simulink model "l8_ver130"  (Simulink 7.9)
settings: Solver=FixedStepDiscrete  SolverMode=SingleTasking  StartTime=0.0  StopTime=10  RelTol=1e-3
undefined symbols (not defined in the file or in its folder): sfp
libraries: xbsIndex_r4 x20466, casper_library_misc x43, dspsigops x32, simulink x7, xps_library x5, casper_library_flow_control x4
(part 22 of 210)

== system l8_ver130/s2/lo_6 ==
  addr : Inport
  pol1 : Inport  Port=2
  pol2 : Inport  Port=3
  Constant10 : xbsIndex_r4/Constant  const=0  gui_display_data_type=Fixed-point  arith_type=Unsigned  n_bits=18  bin_pt=0  preci_type=Single  exp_width=8  frac_width=24  explicit_period=on  period=1  equ=P=C  opselect=C  inp2=PCIN>>17  opr=+  inp1=P  carry=CIN  dbl_ovrd=off  block_type=constant
  Constant9 : xbsIndex_r4/Constant  const=0  gui_display_data_type=Fixed-point  arith_type=Unsigned  n_bits=18  bin_pt=0  preci_type=Single  exp_width=8  frac_width=24  explicit_period=on  period=1  equ=P=C  opselect=C  inp2=PCIN>>17  opr=+  inp1=P  carry=CIN  dbl_ovrd=off  block_type=constant
  Gateway Out : xbsIndex_r4/Gateway Out  inherit_from_input=off  hdl_port=on  timing_constraint=None  locs_specified=off  LOCs={}  UseAsDAC=off  DACChannel='1'  block_type=gatewayout
      settings: infoedit="Gateway out block.  Converts Xilinx fixed point or floating-point type inputs into ouputs of type Simulink integer, double, or fixed point.<br><br>Hardware notes:  In hardware these blocks become top level output ports or are discarded, depending on how they are configured."
  Gateway Out1 : xbsIndex_r4/Gateway Out  inherit_from_input=off  hdl_port=on  timing_constraint=None  locs_specified=off  LOCs={}  UseAsDAC=off  DACChannel='1'  block_type=gatewayout
      settings: infoedit="Gateway out block.  Converts Xilinx fixed point or floating-point type inputs into ouputs of type Simulink integer, double, or fixed point.<br><br>Hardware notes:  In hardware these blocks become top level output ports or are discarded, depending on how they are configured."
  Mult{1..3,16} (4 alike) : xbsIndex_r4/Mult  precision=Full  arith_type="Signed  (2's comp)"  n_bits=16  bin_pt=14  quantization=Truncate  overflow=Wrap  en=off  latency=mult_latency  dbl_ovrd=off  use_behavioral_HDL=off  opt=Speed  use_embedded=on  optimum_pipeline=off  pipeline=on  use_rpm=on  placement_style=Triangular  block_type=mult
      settings: block_version=11.4
  Reinterpret12 : xbsIndex_r4/Reinterpret  force_arith_type=on  arith_type="Signed  (2's comp)"  force_bin_pt=on  bin_pt=15  block_type=reinterpret
  Reinterpret9 : xbsIndex_r4/Reinterpret  force_arith_type=on  arith_type="Signed  (2's comp)"  force_bin_pt=on  bin_pt=15  block_type=reinterpret
      settings: infoedit="Changes signal type without altering the binary representation.   You can changed the signal between signed and unsigned, and relocate the binary point.<br><br>Hardware notes: In hardware this block costs nothing.<br><br>Example:  Suppose the input is 6 bits wide, signed, with 2 fractional bits, and the output is forced to unsigned with 0 fractional bits.  Then an input of -2.0 (1110.00 in binary 2's complement) becomes an output of 56 (111000 in binary)."
  Scope : Scope  NumInputPorts=2
      settings: YMin=-0.0075~-1  YMax=0.0075~1  SaveName=ScopeData3
  cos_slice : xbsIndex_r4/Slice  nbits=16  boolean_output=off  mode=Lower Bit Location + Width  bit1=0  base1=MSB of Input  bit0=0  base0=LSB of Input  dbl_ovrd=off  block_type=slice
  delay{1..3,80} (4 alike) : xbsIndex_r4/Delay  rst=off  infoeditControl=Selection of Reset will increase slice count due to use of real FFs and instead of SRLs  en=off  latency=3  dbl_ovrd=off  reg_retiming=on  block_type=delay
      settings: block_version=11.4
  delay{4..10,32} (8 alike) : xbsIndex_r4/Delay  rst=off  infoeditControl=Selection of Reset will increase slice count due to use of real FFs and instead of SRLs  en=off  latency=4  dbl_ovrd=off  reg_retiming=on  block_type=delay
      settings: block_version=11.4
  lo_ram : Subsystem  Tag=xps:bram  AncestorBlock=xps_library/Shared BRAM  mask{arith_type=Signed  (2's comp), addr_width=bram_length, data_width=32, reg_prim_output=off, reg_core_output=off, optimization=Minimum_Area, data_bin_pt=0, init_vals=[1:2^11], sample_rate=1}
      mask prompts: arith_type "Output Data Type"; addr_width "Address width"; data_width "Data Width"; reg_prim_output "Register Primitive Output"; reg_core_output "Register Core Output"; data_bin_pt "Data Binary Point"; init_vals "Initial values (simulation only)"; sample_rate "Sample rate"
      mask init:
      | shared_bram_mask;
      mask StyleString: popup(Unsigned|Signed  (2's comp)),edit,popup(8|16|32|64|128),checkbox,checkbox,popup(Minimum_Area|Low_Power),edit,edit,edit
  sin_slice : xbsIndex_r4/Slice  nbits=16  boolean_output=off  mode=Upper Bit Location + Width  bit1=0  base1=MSB of Input  bit0=0  base0=LSB of Input  dbl_ovrd=off  block_type=slice
  pol1_im : Outport
  pol2_im : Outport  Port=2
  pol1_re : Outport  Port=3
  pol2_re : Outport  Port=4
  wires:
  Constant9/1 -> lo_ram/3(we)
  Constant10/1 -> lo_ram/2(data_in)
  Mult16/1 -> delay80/1
  Reinterpret12/1 -> Gateway Out/1, delay6/1, delay4/1
  sin_slice/1 -> Reinterpret12/1
  lo_ram/1(data_out) -> cos_slice/1, sin_slice/1
  Mult1/1 -> delay1/1
  Mult2/1 -> delay2/1
  Reinterpret9/1 -> Gateway Out1/1, delay10/1, delay8/1
  cos_slice/1 -> Reinterpret9/1
  Mult3/1 -> delay3/1
  delay32/1 -> Mult16/1
  delay4/1 -> Mult16/2
  delay5/1 -> Mult1/1
  delay6/1 -> Mult1/2
  delay7/1 -> Mult2/1
  delay8/1 -> Mult2/2
  delay9/1 -> Mult3/1
  delay10/1 -> Mult3/2
  delay80/1 -> pol1_im/1
  pol2/1 -> delay5/1, delay9/1
  delay1/1 -> pol2_im/1
  delay2/1 -> pol1_re/1
  delay3/1 -> pol2_re/1
  pol1/1 -> delay32/1, delay7/1
  addr/1 -> lo_ram/1(addr)
  Gateway Out/1 -> Scope/1
  Gateway Out1/1 -> Scope/2

== system l8_ver130/s2/lo_7 ==
  addr : Inport
  pol1 : Inport  Port=2
  pol2 : Inport  Port=3
  Constant10 : xbsIndex_r4/Constant  const=0  gui_display_data_type=Fixed-point  arith_type=Unsigned  n_bits=18  bin_pt=0  preci_type=Single  exp_width=8  frac_width=24  explicit_period=on  period=1  equ=P=C  opselect=C  inp2=PCIN>>17  opr=+  inp1=P  carry=CIN  dbl_ovrd=off  block_type=constant
  Constant9 : xbsIndex_r4/Constant  const=0  gui_display_data_type=Fixed-point  arith_type=Unsigned  n_bits=18  bin_pt=0  preci_type=Single  exp_width=8  frac_width=24  explicit_period=on  period=1  equ=P=C  opselect=C  inp2=PCIN>>17  opr=+  inp1=P  carry=CIN  dbl_ovrd=off  block_type=constant
  Gateway Out : xbsIndex_r4/Gateway Out  inherit_from_input=off  hdl_port=on  timing_constraint=None  locs_specified=off  LOCs={}  UseAsDAC=off  DACChannel='1'  block_type=gatewayout
      settings: infoedit="Gateway out block.  Converts Xilinx fixed point or floating-point type inputs into ouputs of type Simulink integer, double, or fixed point.<br><br>Hardware notes:  In hardware these blocks become top level output ports or are discarded, depending on how they are configured."
  Gateway Out1 : xbsIndex_r4/Gateway Out  inherit_from_input=off  hdl_port=on  timing_constraint=None  locs_specified=off  LOCs={}  UseAsDAC=off  DACChannel='1'  block_type=gatewayout
      settings: infoedit="Gateway out block.  Converts Xilinx fixed point or floating-point type inputs into ouputs of type Simulink integer, double, or fixed point.<br><br>Hardware notes:  In hardware these blocks become top level output ports or are discarded, depending on how they are configured."
  Mult{1..3,16} (4 alike) : xbsIndex_r4/Mult  precision=Full  arith_type="Signed  (2's comp)"  n_bits=16  bin_pt=14  quantization=Truncate  overflow=Wrap  en=off  latency=mult_latency  dbl_ovrd=off  use_behavioral_HDL=off  opt=Speed  use_embedded=on  optimum_pipeline=off  pipeline=on  use_rpm=on  placement_style=Triangular  block_type=mult
      settings: block_version=11.4
  Reinterpret12 : xbsIndex_r4/Reinterpret  force_arith_type=on  arith_type="Signed  (2's comp)"  force_bin_pt=on  bin_pt=15  block_type=reinterpret
  Reinterpret9 : xbsIndex_r4/Reinterpret  force_arith_type=on  arith_type="Signed  (2's comp)"  force_bin_pt=on  bin_pt=15  block_type=reinterpret
      settings: infoedit="Changes signal type without altering the binary representation.   You can changed the signal between signed and unsigned, and relocate the binary point.<br><br>Hardware notes: In hardware this block costs nothing.<br><br>Example:  Suppose the input is 6 bits wide, signed, with 2 fractional bits, and the output is forced to unsigned with 0 fractional bits.  Then an input of -2.0 (1110.00 in binary 2's complement) becomes an output of 56 (111000 in binary)."
  Scope : Scope  NumInputPorts=2
      settings: YMin=-0.0075~-1  YMax=0.0075~1  SaveName=ScopeData3
  cos_slice : xbsIndex_r4/Slice  nbits=16  boolean_output=off  mode=Lower Bit Location + Width  bit1=0  base1=MSB of Input  bit0=0  base0=LSB of Input  dbl_ovrd=off  block_type=slice
  delay{1..3,80} (4 alike) : xbsIndex_r4/Delay  rst=off  infoeditControl=Selection of Reset will increase slice count due to use of real FFs and instead of SRLs  en=off  latency=3  dbl_ovrd=off  reg_retiming=on  block_type=delay
      settings: block_version=11.4
  delay{4..10,32} (8 alike) : xbsIndex_r4/Delay  rst=off  infoeditControl=Selection of Reset will increase slice count due to use of real FFs and instead of SRLs  en=off  latency=4  dbl_ovrd=off  reg_retiming=on  block_type=delay
      settings: block_version=11.4
  lo_ram : Subsystem  Tag=xps:bram  AncestorBlock=xps_library/Shared BRAM  mask{arith_type=Signed  (2's comp), addr_width=bram_length, data_width=32, reg_prim_output=off, reg_core_output=off, optimization=Minimum_Area, data_bin_pt=0, init_vals=[1:2^11], sample_rate=1}
      mask prompts: arith_type "Output Data Type"; addr_width "Address width"; data_width "Data Width"; reg_prim_output "Register Primitive Output"; reg_core_output "Register Core Output"; data_bin_pt "Data Binary Point"; init_vals "Initial values (simulation only)"; sample_rate "Sample rate"
      mask init:
      | shared_bram_mask;
      mask StyleString: popup(Unsigned|Signed  (2's comp)),edit,popup(8|16|32|64|128),checkbox,checkbox,popup(Minimum_Area|Low_Power),edit,edit,edit
  sin_slice : xbsIndex_r4/Slice  nbits=16  boolean_output=off  mode=Upper Bit Location + Width  bit1=0  base1=MSB of Input  bit0=0  base0=LSB of Input  dbl_ovrd=off  block_type=slice
  pol1_im : Outport
  pol2_im : Outport  Port=2
  pol1_re : Outport  Port=3
  pol2_re : Outport  Port=4
  wires:
  Gateway Out1/1 -> Scope/2
  Gateway Out/1 -> Scope/1
  addr/1 -> lo_ram/1(addr)
  pol1/1 -> delay7/1, delay32/1
  delay3/1 -> pol2_re/1
  delay2/1 -> pol1_re/1
  delay1/1 -> pol2_im/1
  pol2/1 -> delay9/1, delay5/1
  delay80/1 -> pol1_im/1
  delay10/1 -> Mult3/2
  delay9/1 -> Mult3/1
  delay8/1 -> Mult2/2
  delay7/1 -> Mult2/1
  delay6/1 -> Mult1/2
  delay5/1 -> Mult1/1
  delay4/1 -> Mult16/2
  delay32/1 -> Mult16/1
  Mult3/1 -> delay3/1
  cos_slice/1 -> Reinterpret9/1
  Reinterpret9/1 -> delay8/1, delay10/1, Gateway Out1/1
  Mult2/1 -> delay2/1
  Mult1/1 -> delay1/1
  lo_ram/1(data_out) -> sin_slice/1, cos_slice/1
  sin_slice/1 -> Reinterpret12/1
  Reinterpret12/1 -> delay4/1, delay6/1, Gateway Out/1
  Mult16/1 -> delay80/1
  Constant10/1 -> lo_ram/2(data_in)
  Constant9/1 -> lo_ram/3(we)

== system l8_ver130/s2/lo_8 ==
  addr : Inport
  pol1 : Inport  Port=2
  pol2 : Inport  Port=3
  Constant10 : xbsIndex_r4/Constant  const=0  gui_display_data_type=Fixed-point  arith_type=Unsigned  n_bits=18  bin_pt=0  preci_type=Single  exp_width=8  frac_width=24  explicit_period=on  period=1  equ=P=C  opselect=C  inp2=PCIN>>17  opr=+  inp1=P  carry=CIN  dbl_ovrd=off  block_type=constant
  Constant9 : xbsIndex_r4/Constant  const=0  gui_display_data_type=Fixed-point  arith_type=Unsigned  n_bits=18  bin_pt=0  preci_type=Single  exp_width=8  frac_width=24  explicit_period=on  period=1  equ=P=C  opselect=C  inp2=PCIN>>17  opr=+  inp1=P  carry=CIN  dbl_ovrd=off  block_type=constant
  Gateway Out : xbsIndex_r4/Gateway Out  inherit_from_input=off  hdl_port=on  timing_constraint=None  locs_specified=off  LOCs={}  UseAsDAC=off  DACChannel='1'  block_type=gatewayout
      settings: infoedit="Gateway out block.  Converts Xilinx fixed point or floating-point type inputs into ouputs of type Simulink integer, double, or fixed point.<br><br>Hardware notes:  In hardware these blocks become top level output ports or are discarded, depending on how they are configured."
  Gateway Out1 : xbsIndex_r4/Gateway Out  inherit_from_input=off  hdl_port=on  timing_constraint=None  locs_specified=off  LOCs={}  UseAsDAC=off  DACChannel='1'  block_type=gatewayout
      settings: infoedit="Gateway out block.  Converts Xilinx fixed point or floating-point type inputs into ouputs of type Simulink integer, double, or fixed point.<br><br>Hardware notes:  In hardware these blocks become top level output ports or are discarded, depending on how they are configured."
  Mult{1..3,16} (4 alike) : xbsIndex_r4/Mult  precision=Full  arith_type="Signed  (2's comp)"  n_bits=16  bin_pt=14  quantization=Truncate  overflow=Wrap  en=off  latency=mult_latency  dbl_ovrd=off  use_behavioral_HDL=off  opt=Speed  use_embedded=on  optimum_pipeline=off  pipeline=on  use_rpm=on  placement_style=Triangular  block_type=mult
      settings: block_version=11.4
  Reinterpret12 : xbsIndex_r4/Reinterpret  force_arith_type=on  arith_type="Signed  (2's comp)"  force_bin_pt=on  bin_pt=15  block_type=reinterpret
  Reinterpret9 : xbsIndex_r4/Reinterpret  force_arith_type=on  arith_type="Signed  (2's comp)"  force_bin_pt=on  bin_pt=15  block_type=reinterpret
      settings: infoedit="Changes signal type without altering the binary representation.   You can changed the signal between signed and unsigned, and relocate the binary point.<br><br>Hardware notes: In hardware this block costs nothing.<br><br>Example:  Suppose the input is 6 bits wide, signed, with 2 fractional bits, and the output is forced to unsigned with 0 fractional bits.  Then an input of -2.0 (1110.00 in binary 2's complement) becomes an output of 56 (111000 in binary)."
  Scope : Scope  NumInputPorts=2
      settings: YMin=-0.0075~-1  YMax=0.0075~1  SaveName=ScopeData3
  cos_slice : xbsIndex_r4/Slice  nbits=16  boolean_output=off  mode=Lower Bit Location + Width  bit1=0  base1=MSB of Input  bit0=0  base0=LSB of Input  dbl_ovrd=off  block_type=slice
  delay{1..3,80} (4 alike) : xbsIndex_r4/Delay  rst=off  infoeditControl=Selection of Reset will increase slice count due to use of real FFs and instead of SRLs  en=off  latency=3  dbl_ovrd=off  reg_retiming=on  block_type=delay
      settings: block_version=11.4
  delay{4..10,32} (8 alike) : xbsIndex_r4/Delay  rst=off  infoeditControl=Selection of Reset will increase slice count due to use of real FFs and instead of SRLs  en=off  latency=4  dbl_ovrd=off  reg_retiming=on  block_type=delay
      settings: block_version=11.4
  lo_ram : Subsystem  Tag=xps:bram  AncestorBlock=xps_library/Shared BRAM  mask{arith_type=Signed  (2's comp), addr_width=bram_length, data_width=32, reg_prim_output=off, reg_core_output=off, optimization=Minimum_Area, data_bin_pt=0, init_vals=[1:2^11], sample_rate=1}
      mask prompts: arith_type "Output Data Type"; addr_width "Address width"; data_width "Data Width"; reg_prim_output "Register Primitive Output"; reg_core_output "Register Core Output"; data_bin_pt "Data Binary Point"; init_vals "Initial values (simulation only)"; sample_rate "Sample rate"
      mask init:
      | shared_bram_mask;
      mask StyleString: popup(Unsigned|Signed  (2's comp)),edit,popup(8|16|32|64|128),checkbox,checkbox,popup(Minimum_Area|Low_Power),edit,edit,edit
  sin_slice : xbsIndex_r4/Slice  nbits=16  boolean_output=off  mode=Upper Bit Location + Width  bit1=0  base1=MSB of Input  bit0=0  base0=LSB of Input  dbl_ovrd=off  block_type=slice
  pol1_im : Outport
  pol2_im : Outport  Port=2
  pol1_re : Outport  Port=3
  pol2_re : Outport  Port=4
  wires:
  Constant9/1 -> lo_ram/3(we)
  Constant10/1 -> lo_ram/2(data_in)
  Mult16/1 -> delay80/1
  Reinterpret12/1 -> Gateway Out/1, delay6/1, delay4/1
  sin_slice/1 -> Reinterpret12/1
  lo_ram/1(data_out) -> cos_slice/1, sin_slice/1
  Mult1/1 -> delay1/1
  Mult2/1 -> delay2/1
  Reinterpret9/1 -> Gateway Out1/1, delay10/1, delay8/1
  cos_slice/1 -> Reinterpret9/1
  Mult3/1 -> delay3/1
  delay32/1 -> Mult16/1
  delay4/1 -> Mult16/2
  delay5/1 -> Mult1/1
  delay6/1 -> Mult1/2
  delay7/1 -> Mult2/1
  delay8/1 -> Mult2/2
  delay9/1 -> Mult3/1
  delay10/1 -> Mult3/2
  delay80/1 -> pol1_im/1
  pol2/1 -> delay5/1, delay9/1
  delay1/1 -> pol2_im/1
  delay2/1 -> pol1_re/1
  delay3/1 -> pol2_re/1
  pol1/1 -> delay32/1, delay7/1
  addr/1 -> lo_ram/1(addr)
  Gateway Out/1 -> Scope/1
  Gateway Out1/1 -> Scope/2

== system l8_ver130/s2/lo_9 ==
  addr : Inport
  pol1 : Inport  Port=2
  pol2 : Inport  Port=3
  Constant10 : xbsIndex_r4/Constant  const=0  gui_display_data_type=Fixed-point  arith_type=Unsigned  n_bits=18  bin_pt=0  preci_type=Single  exp_width=8  frac_width=24  explicit_period=on  period=1  equ=P=C  opselect=C  inp2=PCIN>>17  opr=+  inp1=P  carry=CIN  dbl_ovrd=off  block_type=constant
  Constant9 : xbsIndex_r4/Constant  const=0  gui_display_data_type=Fixed-point  arith_type=Unsigned  n_bits=18  bin_pt=0  preci_type=Single  exp_width=8  frac_width=24  explicit_period=on  period=1  equ=P=C  opselect=C  inp2=PCIN>>17  opr=+  inp1=P  carry=CIN  dbl_ovrd=off  block_type=constant
  Gateway Out : xbsIndex_r4/Gateway Out  inherit_from_input=off  hdl_port=on  timing_constraint=None  locs_specified=off  LOCs={}  UseAsDAC=off  DACChannel='1'  block_type=gatewayout
      settings: infoedit="Gateway out block.  Converts Xilinx fixed point or floating-point type inputs into ouputs of type Simulink integer, double, or fixed point.<br><br>Hardware notes:  In hardware these blocks become top level output ports or are discarded, depending on how they are configured."
  Gateway Out1 : xbsIndex_r4/Gateway Out  inherit_from_input=off  hdl_port=on  timing_constraint=None  locs_specified=off  LOCs={}  UseAsDAC=off  DACChannel='1'  block_type=gatewayout
      settings: infoedit="Gateway out block.  Converts Xilinx fixed point or floating-point type inputs into ouputs of type Simulink integer, double, or fixed point.<br><br>Hardware notes:  In hardware these blocks become top level output ports or are discarded, depending on how they are configured."
  Mult{1..3,16} (4 alike) : xbsIndex_r4/Mult  precision=Full  arith_type="Signed  (2's comp)"  n_bits=16  bin_pt=14  quantization=Truncate  overflow=Wrap  en=off  latency=mult_latency  dbl_ovrd=off  use_behavioral_HDL=off  opt=Speed  use_embedded=on  optimum_pipeline=off  pipeline=on  use_rpm=on  placement_style=Triangular  block_type=mult
      settings: block_version=11.4
  Reinterpret12 : xbsIndex_r4/Reinterpret  force_arith_type=on  arith_type="Signed  (2's comp)"  force_bin_pt=on  bin_pt=15  block_type=reinterpret
  Reinterpret9 : xbsIndex_r4/Reinterpret  force_arith_type=on  arith_type="Signed  (2's comp)"  force_bin_pt=on  bin_pt=15  block_type=reinterpret
      settings: infoedit="Changes signal type without altering the binary representation.   You can changed the signal between signed and unsigned, and relocate the binary point.<br><br>Hardware notes: In hardware this block costs nothing.<br><br>Example:  Suppose the input is 6 bits wide, signed, with 2 fractional bits, and the output is forced to unsigned with 0 fractional bits.  Then an input of -2.0 (1110.00 in binary 2's complement) becomes an output of 56 (111000 in binary)."
  Scope : Scope  NumInputPorts=2
      settings: YMin=-0.0075~-1  YMax=0.0075~1  SaveName=ScopeData3
  cos_slice : xbsIndex_r4/Slice  nbits=16  boolean_output=off  mode=Lower Bit Location + Width  bit1=0  base1=MSB of Input  bit0=0  base0=LSB of Input  dbl_ovrd=off  block_type=slice
  delay{1..3,80} (4 alike) : xbsIndex_r4/Delay  rst=off  infoeditControl=Selection of Reset will increase slice count due to use of real FFs and instead of SRLs  en=off  latency=3  dbl_ovrd=off  reg_retiming=on  block_type=delay
      settings: block_version=11.4
  delay{4..10,32} (8 alike) : xbsIndex_r4/Delay  rst=off  infoeditControl=Selection of Reset will increase slice count due to use of real FFs and instead of SRLs  en=off  latency=4  dbl_ovrd=off  reg_retiming=on  block_type=delay
      settings: block_version=11.4
  lo_ram : Subsystem  Tag=xps:bram  AncestorBlock=xps_library/Shared BRAM  mask{arith_type=Signed  (2's comp), addr_width=bram_length, data_width=32, reg_prim_output=off, reg_core_output=off, optimization=Minimum_Area, data_bin_pt=0, init_vals=[1:2^11], sample_rate=1}
      mask prompts: arith_type "Output Data Type"; addr_width "Address width"; data_width "Data Width"; reg_prim_output "Register Primitive Output"; reg_core_output "Register Core Output"; data_bin_pt "Data Binary Point"; init_vals "Initial values (simulation only)"; sample_rate "Sample rate"
      mask init:
      | shared_bram_mask;
      mask StyleString: popup(Unsigned|Signed  (2's comp)),edit,popup(8|16|32|64|128),checkbox,checkbox,popup(Minimum_Area|Low_Power),edit,edit,edit
  sin_slice : xbsIndex_r4/Slice  nbits=16  boolean_output=off  mode=Upper Bit Location + Width  bit1=0  base1=MSB of Input  bit0=0  base0=LSB of Input  dbl_ovrd=off  block_type=slice
  pol1_im : Outport
  pol2_im : Outport  Port=2
  pol1_re : Outport  Port=3
  pol2_re : Outport  Port=4
  wires:
  Gateway Out1/1 -> Scope/2
  Gateway Out/1 -> Scope/1
  addr/1 -> lo_ram/1(addr)
  pol1/1 -> delay7/1, delay32/1
  delay3/1 -> pol2_re/1
  delay2/1 -> pol1_re/1
  delay1/1 -> pol2_im/1
  pol2/1 -> delay9/1, delay5/1
  delay80/1 -> pol1_im/1
  delay10/1 -> Mult3/2
  delay9/1 -> Mult3/1
  delay8/1 -> Mult2/2
  delay7/1 -> Mult2/1
  delay6/1 -> Mult1/2
  delay5/1 -> Mult1/1
  delay4/1 -> Mult16/2
  delay32/1 -> Mult16/1
  Mult3/1 -> delay3/1
  cos_slice/1 -> Reinterpret9/1
  Reinterpret9/1 -> delay8/1, delay10/1, Gateway Out1/1
  Mult2/1 -> delay2/1
  Mult1/1 -> delay1/1
  lo_ram/1(data_out) -> sin_slice/1, cos_slice/1
  sin_slice/1 -> Reinterpret12/1
  Reinterpret12/1 -> delay4/1, delay6/1, Gateway Out/1
  Mult16/1 -> delay80/1
  Constant10/1 -> lo_ram/2(data_in)
  Constant9/1 -> lo_ram/3(we)

== system l8_ver130/s2/mixer_cnt ==
  sim_reg : Inport
  io_delay : xbsIndex_r4/Delay  rst=off  infoeditControl=Selection of Reset will increase slice count due to use of real FFs and instead of SRLs  en=off  latency=0  dbl_ovrd=off  reg_retiming=on  block_type=delay
  l8_ver130_s2_mixer_cnt_user_data_out : xbsIndex_r4/Gateway In  gui_display_data_type=Fixed-point  arith_type=Unsigned  n_bits=32  bin_pt=0  preci_type=Single  exp_width=8  frac_width=24  quantization="Round  (unbiased: +/- Inf)"  overflow=Saturate  period=1  dbl_ovrd=off  timing_constraint=None  locs_specified=off  LOCs={}  UseAsADC=off  ADCChannel='1'  inherit_from_input=off  hdl_port=on  block_type=gatewayin
  reint1 : xbsIndex_r4/Reinterpret  force_arith_type=on  arith_type=Unsigned  force_bin_pt=on  bin_pt=0  block_type=reinterpret
  slice_reg : xbsIndex_r4/Slice  nbits=32  boolean_output=off  mode=Lower Bit Location + Width  bit1=0  base1=MSB of Input  bit0=0  base0=LSB of Input  dbl_ovrd=off  block_type=slice
  in_reg : Outport
  wires:
  l8_ver130_s2_mixer_cnt_user_data_out/1 -> io_delay/1
  sim_reg/1 -> l8_ver130_s2_mixer_cnt_user_data_out/1
  io_delay/1 -> slice_reg/1
  slice_reg/1 -> reint1/1
  reint1/1 -> in_reg/1

== system l8_ver130/s2/quant ==
  In2 : Inport
  In3 : Inport  Port=2
  In4 : Inport  Port=3
  In5 : Inport  Port=4
  Constant : Constant
  Delay{,2,4,6,8} (5 alike) : xbsIndex_r4/Delay  rst=off  infoeditControl=Selection of Reset will increase slice count due to use of real FFs and instead of SRLs  en=off  latency=5  dbl_ovrd=off  reg_retiming=off  block_type=delay
  Delay{1,3,5,7} (4 alike) : xbsIndex_r4/Delay  rst=off  infoeditControl=Selection of Reset will increase slice count due to use of real FFs and instead of SRLs  en=off  latency=1  dbl_ovrd=off  reg_retiming=off  block_type=delay
  gain : Subsystem  Tag=xps:sw_reg  AncestorBlock=xps_library/software register  mask{numios=1, io_dir=From Processor, io_delay=0, sample_period=1, name1=reg, bitwidth1=32, arith_type1=Unsigned, bin_pt1=0}
      mask prompts: numios "Num I/Os"; io_dir "I/O direction"; io_delay "I/O delay"; sample_period "Sample period"; name1 "Name"; bitwidth1 "Bitwidth"; arith_type1 "Type"; bin_pt1 "Binary pt"
      mask init:
      | swreg_init(gcb);
      mask StyleString: edit,popup(To Processor|From Processor),edit,edit,edit,edit,popup(Unsigned|Signed  (2's comp)|Boolean),edit
      mask CallbackString:
      | t = timer('StartDelay', 0.1); t.TimerFcn = {@swreg_cb, gcb}; start(t);|a = get_param(gcb, 'MaskEnables');
      | if strcmp(get_param(gcb, 'io_dir'), 'To Processor'),
      | a(4) = {'off'};
      | else
      | a(4) = {'on'};
      | end
      | set_param(gcb, 'MaskEnables', a);
      | |||||a = get_param(gcb, 'MaskEnables');
      | if strcmp(get_param(gcb, 'arith_type1'), 'Boolean'),
      | set_param(gcb, 'bitwidth1', '1');
      | set_param(gcb, 'bin_pt1', '0');
      | a(6) = {'off'};
      | a(8) = {'off'};
      | else
      | a(6) = {'on'};
      | a(8) = {'on'};
      | end
      | set_param(gcb, 'MaskEnables', a);
      | |
      mask TabNameString: Setup,Setup,Setup,Setup,IO1,IO1,IO1,IO1
  round : Subsystem
  round1 : Subsystem
  round2 : Subsystem
  round3 : Subsystem
  Out1 : Outport
  Out2 : Outport  Port=2
  Out3 : Outport  Port=3
  Out4 : Outport  Port=4
  wires:
  Delay1/1 -> Delay/1
  Delay3/1 -> Delay2/1
  Delay5/1 -> Delay4/1
  Delay7/1 -> Delay6/1
  Delay/1 -> round/1(dIn)
  Delay2/1 -> round1/1(dIn)
  Delay4/1 -> round2/1(dIn)
  Delay6/1 -> round3/1(dIn)
  gain/1(in_reg) -> Delay8/1
  Delay8/1 -> round/2(Scale), round1/2(Scale), round2/2(Scale), round3/2(Scale)
  In2/1 -> Delay1/1
  round/1(dOut) -> Out1/1
  In3/1 -> Delay3/1
  round1/1(dOut) -> Out2/1
  In4/1 -> Delay5/1
  round2/1(dOut) -> Out3/1
  In5/1 -> Delay7/1
  round3/1(dOut) -> Out4/1
  Constant/1 -> gain/1(sim_reg)

== system l8_ver130/s3/Halfband0 ==
  in : Inport
  sync : Inport  Port=2
  Delay{1..14} (14 alike) : xbsIndex_r4/Delay  rst=off  infoeditControl=Selection of Reset will increase slice count due to use of real FFs and instead of SRLs  en=off  latency=2  dbl_ovrd=off  reg_retiming=off  block_type=delay
  adder_tree : Subsystem
      settings: AttributesFormatString=|
      | latency 4
      | hold period:2
  en_gen : Subsystem
      settings: AttributesFormatString=|
      | hold period: 2
      | explicit clk period:off , input clk period:1
      | relational/logical delay:1
      | extra_delay:0
  hold_fir_tap1 : Subsystem
      settings: AttributesFormatString=|
      | coefficient: -0.026473
      |  hold period: 2
      |  mult latency: 3
  hold_fir_tap11 : Subsystem
      settings: AttributesFormatString=|
      | coefficient: -0.093407
      |  hold period: 2
      |  mult latency: 3
  hold_fir_tap13 : Subsystem
      settings: AttributesFormatString=|
      | coefficient: 0.044090
      |  hold period: 2
      |  mult latency: 3
  hold_fir_tap15 : Subsystem
      settings: AttributesFormatString=|
      | coefficient: -0.026473
      |  hold period: 2
      |  mult latency: 3
  hold_fir_tap3 : Subsystem
      settings: AttributesFormatString=|
      | coefficient: 0.044090
      |  hold period: 2
      |  mult latency: 3
  hold_fir_tap5 : Subsystem
      settings: AttributesFormatString=|
      | coefficient: -0.093407
      |  hold period: 2
      |  mult latency: 3
  hold_fir_tap7 : Subsystem
      settings: AttributesFormatString=|
      | coefficient: 0.313910
      |  hold period: 2
      |  mult latency: 3
  hold_fir_tap8 : Subsystem
      settings: AttributesFormatString=|
      | coefficient: 0.500000
      |  hold period: 2
      |  mult latency: 3
  hold_fir_tap9 : Subsystem
      settings: AttributesFormatString=|
      | coefficient: 0.313910
      |  hold period: 2
      |  mult latency: 3
  out : Outport
  en_out : Outport  Port=2
  wires:
  in/1 -> hold_fir_tap1/1(data_in), Delay1/1
  Delay2/1 -> hold_fir_tap3/1(data_in), Delay3/1  "xlsub2_Delay_out2"
  Delay4/1 -> hold_fir_tap5/1(data_in), Delay5/1  "xlsub2_Delay_out4"
  Delay6/1 -> hold_fir_tap7/1(data_in), Delay7/1  "xlsub2_Delay_out6"
  Delay7/1 -> hold_fir_tap8/1(data_in), Delay8/1  "xlsub2_Delay_out7"
  Delay8/1 -> hold_fir_tap9/1(data_in), Delay9/1  "xlsub2_Delay_out8"
  Delay10/1 -> hold_fir_tap11/1(data_in), Delay11/1  "xlsub2_Delay_out10"
  Delay12/1 -> hold_fir_tap13/1(data_in), Delay13/1  "xlsub2_Delay_out12"
  en_gen/1(en_out) -> adder_tree/1(en_in), hold_fir_tap15/2(en_in), hold_fir_tap13/2(en_in), hold_fir_tap11/2(en_in), hold_fir_tap9/2(en_in), hold_fir_tap8/2(en_in), hold_fir_tap7/2(en_in), hold_fir_tap5/2(en_in), hold_fir_tap3/2(en_in), hold_fir_tap1/2(en_in)  "en"
  adder_tree/2(dout) -> out/1
  adder_tree/1(en_out) -> en_out/1
  hold_fir_tap15/1(mult_out) -> adder_tree/10(din9)  "hold_fir_tap_out9"
  hold_fir_tap13/1(mult_out) -> adder_tree/9(din8)  "hold_fir_tap_out8"
  hold_fir_tap11/1(mult_out) -> adder_tree/8(din7)  "hold_fir_tap_out7"
  hold_fir_tap9/1(mult_out) -> adder_tree/7(din6)  "hold_fir_tap_out6"
  hold_fir_tap8/1(mult_out) -> adder_tree/6(din5)  "hold_fir_tap_out5"
  hold_fir_tap7/1(mult_out) -> adder_tree/5(din4)  "hold_fir_tap_out4"
  hold_fir_tap5/1(mult_out) -> adder_tree/4(din3)  "hold_fir_tap_out3"
  hold_fir_tap3/1(mult_out) -> adder_tree/3(din2)  "hold_fir_tap_out2"
  hold_fir_tap1/1(mult_out) -> adder_tree/2(din1)  "hold_fir_tap_out1"
  Delay14/1 -> hold_fir_tap15/1(data_in)  "xlsub2_Delay_out14"
  Delay13/1 -> Delay14/1  "xlsub2_Delay_out13"
  Delay11/1 -> Delay12/1  "xlsub2_Delay_out11"
  Delay9/1 -> Delay10/1  "xlsub2_Delay_out9"
  Delay5/1 -> Delay6/1  "xlsub2_Delay_out5"
  Delay3/1 -> Delay4/1  "xlsub2_Delay_out3"
  Delay1/1 -> Delay2/1  "xlsub2_Delay_out1"
  sync/1 -> en_gen/1(sync_in)

== system l8_ver130/s3/Halfband1 ==
  in : Inport
  sync : Inport  Port=2
  Delay{1..14} (14 alike) : xbsIndex_r4/Delay  rst=off  infoeditControl=Selection of Reset will increase slice count due to use of real FFs and instead of SRLs  en=off  latency=2  dbl_ovrd=off  reg_retiming=off  block_type=delay
  adder_tree : Subsystem
      settings: AttributesFormatString=|
      | latency 4
      | hold period:2
  en_gen : Subsystem
      settings: AttributesFormatString=|
      | hold period: 2
      | explicit clk period:off , input clk period:1
      | relational/logical delay:1
      | extra_delay:0
  hold_fir_tap1 : Subsystem
      settings: AttributesFormatString=|
      | coefficient: -0.026473
      |  hold period: 2
      |  mult latency: 3
  hold_fir_tap11 : Subsystem
      settings: AttributesFormatString=|
      | coefficient: -0.093407
      |  hold period: 2
      |  mult latency: 3
  hold_fir_tap13 : Subsystem
      settings: AttributesFormatString=|
      | coefficient: 0.044090
      |  hold period: 2
      |  mult latency: 3
  hold_fir_tap15 : Subsystem
      settings: AttributesFormatString=|
      | coefficient: -0.026473
      |  hold period: 2
      |  mult latency: 3
  hold_fir_tap3 : Subsystem
      settings: AttributesFormatString=|
      | coefficient: 0.044090
      |  hold period: 2
      |  mult latency: 3
  hold_fir_tap5 : Subsystem
      settings: AttributesFormatString=|
      | coefficient: -0.093407
      |  hold period: 2
      |  mult latency: 3
  hold_fir_tap7 : Subsystem
      settings: AttributesFormatString=|
      | coefficient: 0.313910
      |  hold period: 2
      |  mult latency: 3
  hold_fir_tap8 : Subsystem
      settings: AttributesFormatString=|
      | coefficient: 0.500000
      |  hold period: 2
      |  mult latency: 3
  hold_fir_tap9 : Subsystem
      settings: AttributesFormatString=|
      | coefficient: 0.313910
      |  hold period: 2
      |  mult latency: 3
  out : Outport
  en_out : Outport  Port=2
  wires:
  in/1 -> hold_fir_tap1/1(data_in), Delay1/1
  Delay2/1 -> hold_fir_tap3/1(data_in), Delay3/1  "xlsub2_Delay_out2"
  Delay4/1 -> hold_fir_tap5/1(data_in), Delay5/1  "xlsub2_Delay_out4"
  Delay6/1 -> hold_fir_tap7/1(data_in), Delay7/1  "xlsub2_Delay_out6"
  Delay7/1 -> hold_fir_tap8/1(data_in), Delay8/1  "xlsub2_Delay_out7"
  Delay8/1 -> hold_fir_tap9/1(data_in), Delay9/1  "xlsub2_Delay_out8"
  Delay10/1 -> hold_fir_tap11/1(data_in), Delay11/1  "xlsub2_Delay_out10"
  Delay12/1 -> hold_fir_tap13/1(data_in), Delay13/1  "xlsub2_Delay_out12"
  en_gen/1(en_out) -> adder_tree/1(en_in), hold_fir_tap15/2(en_in), hold_fir_tap13/2(en_in), hold_fir_tap11/2(en_in), hold_fir_tap9/2(en_in), hold_fir_tap8/2(en_in), hold_fir_tap7/2(en_in), hold_fir_tap5/2(en_in), hold_fir_tap3/2(en_in), hold_fir_tap1/2(en_in)  "en"
  adder_tree/2(dout) -> out/1
  adder_tree/1(en_out) -> en_out/1
  hold_fir_tap15/1(mult_out) -> adder_tree/10(din9)  "hold_fir_tap_out9"
  hold_fir_tap13/1(mult_out) -> adder_tree/9(din8)  "hold_fir_tap_out8"
  hold_fir_tap11/1(mult_out) -> adder_tree/8(din7)  "hold_fir_tap_out7"
  hold_fir_tap9/1(mult_out) -> adder_tree/7(din6)  "hold_fir_tap_out6"
  hold_fir_tap8/1(mult_out) -> adder_tree/6(din5)  "hold_fir_tap_out5"
  hold_fir_tap7/1(mult_out) -> adder_tree/5(din4)  "hold_fir_tap_out4"
  hold_fir_tap5/1(mult_out) -> adder_tree/4(din3)  "hold_fir_tap_out3"
  hold_fir_tap3/1(mult_out) -> adder_tree/3(din2)  "hold_fir_tap_out2"
  hold_fir_tap1/1(mult_out) -> adder_tree/2(din1)  "hold_fir_tap_out1"
  Delay14/1 -> hold_fir_tap15/1(data_in)  "xlsub2_Delay_out14"
  Delay13/1 -> Delay14/1  "xlsub2_Delay_out13"
  Delay11/1 -> Delay12/1  "xlsub2_Delay_out11"
  Delay9/1 -> Delay10/1  "xlsub2_Delay_out9"
  Delay5/1 -> Delay6/1  "xlsub2_Delay_out5"
  Delay3/1 -> Delay4/1  "xlsub2_Delay_out3"
  Delay1/1 -> Delay2/1  "xlsub2_Delay_out1"
  sync/1 -> en_gen/1(sync_in)

== system l8_ver130/s3/Halfband2 ==
  in : Inport
  sync : Inport  Port=2
  Delay{1..14} (14 alike) : xbsIndex_r4/Delay  rst=off  infoeditControl=Selection of Reset will increase slice count due to use of real FFs and instead of SRLs  en=off  latency=2  dbl_ovrd=off  reg_retiming=off  block_type=delay
  adder_tree : Subsystem
      settings: AttributesFormatString=|
      | latency 4
      | hold period:2
  en_gen : Subsystem
      settings: AttributesFormatString=|
      | hold period: 2
      | explicit clk period:off , input clk period:1
      | relational/logical delay:1
      | extra_delay:0
  hold_fir_tap1 : Subsystem
      settings: AttributesFormatString=|
      | coefficient: -0.026473
      |  hold period: 2
      |  mult latency: 3
  hold_fir_tap11 : Subsystem
      settings: AttributesFormatString=|
      | coefficient: -0.093407
      |  hold period: 2
      |  mult latency: 3
  hold_fir_tap13 : Subsystem
      settings: AttributesFormatString=|
      | coefficient: 0.044090
      |  hold period: 2
      |  mult latency: 3
  hold_fir_tap15 : Subsystem
      settings: AttributesFormatString=|
      | coefficient: -0.026473
      |  hold period: 2
      |  mult latency: 3
  hold_fir_tap3 : Subsystem
      settings: AttributesFormatString=|
      | coefficient: 0.044090
      |  hold period: 2
      |  mult latency: 3
  hold_fir_tap5 : Subsystem
      settings: AttributesFormatString=|
      | coefficient: -0.093407
      |  hold period: 2
      |  mult latency: 3
  hold_fir_tap7 : Subsystem
      settings: AttributesFormatString=|
      | coefficient: 0.313910
      |  hold period: 2
      |  mult latency: 3
  hold_fir_tap8 : Subsystem
      settings: AttributesFormatString=|
      | coefficient: 0.500000
      |  hold period: 2
      |  mult latency: 3
  hold_fir_tap9 : Subsystem
      settings: AttributesFormatString=|
      | coefficient: 0.313910
      |  hold period: 2
      |  mult latency: 3
  out : Outport
  en_out : Outport  Port=2
  wires:
  in/1 -> hold_fir_tap1/1(data_in), Delay1/1
  Delay2/1 -> hold_fir_tap3/1(data_in), Delay3/1  "xlsub2_Delay_out2"
  Delay4/1 -> hold_fir_tap5/1(data_in), Delay5/1  "xlsub2_Delay_out4"
  Delay6/1 -> hold_fir_tap7/1(data_in), Delay7/1  "xlsub2_Delay_out6"
  Delay7/1 -> hold_fir_tap8/1(data_in), Delay8/1  "xlsub2_Delay_out7"
  Delay8/1 -> hold_fir_tap9/1(data_in), Delay9/1  "xlsub2_Delay_out8"
  Delay10/1 -> hold_fir_tap11/1(data_in), Delay11/1  "xlsub2_Delay_out10"
  Delay12/1 -> hold_fir_tap13/1(data_in), Delay13/1  "xlsub2_Delay_out12"
  en_gen/1(en_out) -> adder_tree/1(en_in), hold_fir_tap15/2(en_in), hold_fir_tap13/2(en_in), hold_fir_tap11/2(en_in), hold_fir_tap9/2(en_in), hold_fir_tap8/2(en_in), hold_fir_tap7/2(en_in), hold_fir_tap5/2(en_in), hold_fir_tap3/2(en_in), hold_fir_tap1/2(en_in)  "en"
  adder_tree/2(dout) -> out/1
  adder_tree/1(en_out) -> en_out/1
  hold_fir_tap15/1(mult_out) -> adder_tree/10(din9)  "hold_fir_tap_out9"
  hold_fir_tap13/1(mult_out) -> adder_tree/9(din8)  "hold_fir_tap_out8"
  hold_fir_tap11/1(mult_out) -> adder_tree/8(din7)  "hold_fir_tap_out7"
  hold_fir_tap9/1(mult_out) -> adder_tree/7(din6)  "hold_fir_tap_out6"
  hold_fir_tap8/1(mult_out) -> adder_tree/6(din5)  "hold_fir_tap_out5"
  hold_fir_tap7/1(mult_out) -> adder_tree/5(din4)  "hold_fir_tap_out4"
  hold_fir_tap5/1(mult_out) -> adder_tree/4(din3)  "hold_fir_tap_out3"
  hold_fir_tap3/1(mult_out) -> adder_tree/3(din2)  "hold_fir_tap_out2"
  hold_fir_tap1/1(mult_out) -> adder_tree/2(din1)  "hold_fir_tap_out1"
  Delay14/1 -> hold_fir_tap15/1(data_in)  "xlsub2_Delay_out14"
  Delay13/1 -> Delay14/1  "xlsub2_Delay_out13"
  Delay11/1 -> Delay12/1  "xlsub2_Delay_out11"
  Delay9/1 -> Delay10/1  "xlsub2_Delay_out9"
  Delay5/1 -> Delay6/1  "xlsub2_Delay_out5"
  Delay3/1 -> Delay4/1  "xlsub2_Delay_out3"
  Delay1/1 -> Delay2/1  "xlsub2_Delay_out1"
  sync/1 -> en_gen/1(sync_in)

== system l8_ver130/s3/Halfband3 ==
  in : Inport
  sync : Inport  Port=2
  Delay{1..14} (14 alike) : xbsIndex_r4/Delay  rst=off  infoeditControl=Selection of Reset will increase slice count due to use of real FFs and instead of SRLs  en=off  latency=2  dbl_ovrd=off  reg_retiming=off  block_type=delay
  adder_tree : Subsystem
      settings: AttributesFormatString=|
      | latency 4
      | hold period:2
  en_gen : Subsystem
      settings: AttributesFormatString=|
      | hold period: 2
      | explicit clk period:off , input clk period:1
      | relational/logical delay:1
      | extra_delay:0
  hold_fir_tap1 : Subsystem
      settings: AttributesFormatString=|
      | coefficient: -0.026473
      |  hold period: 2
      |  mult latency: 3
  hold_fir_tap11 : Subsystem
      settings: AttributesFormatString=|
      | coefficient: -0.093407
      |  hold period: 2
      |  mult latency: 3
  hold_fir_tap13 : Subsystem
      settings: AttributesFormatString=|
      | coefficient: 0.044090
      |  hold period: 2
      |  mult latency: 3
  hold_fir_tap15 : Subsystem
      settings: AttributesFormatString=|
      | coefficient: -0.026473
      |  hold period: 2
      |  mult latency: 3
  hold_fir_tap3 : Subsystem
      settings: AttributesFormatString=|
      | coefficient: 0.044090
      |  hold period: 2
      |  mult latency: 3
  hold_fir_tap5 : Subsystem
      settings: AttributesFormatString=|
      | coefficient: -0.093407
      |  hold period: 2
      |  mult latency: 3
  hold_fir_tap7 : Subsystem
      settings: AttributesFormatString=|
      | coefficient: 0.313910
      |  hold period: 2
      |  mult latency: 3
  hold_fir_tap8 : Subsystem
      settings: AttributesFormatString=|
      | coefficient: 0.500000
      |  hold period: 2
      |  mult latency: 3
  hold_fir_tap9 : Subsystem
      settings: AttributesFormatString=|
      | coefficient: 0.313910
      |  hold period: 2
      |  mult latency: 3
  out : Outport
  en_out : Outport  Port=2
  wires:
  in/1 -> hold_fir_tap1/1(data_in), Delay1/1
  Delay2/1 -> hold_fir_tap3/1(data_in), Delay3/1  "xlsub2_Delay_out2"
  Delay4/1 -> hold_fir_tap5/1(data_in), Delay5/1  "xlsub2_Delay_out4"
  Delay6/1 -> hold_fir_tap7/1(data_in), Delay7/1  "xlsub2_Delay_out6"
  Delay7/1 -> hold_fir_tap8/1(data_in), Delay8/1  "xlsub2_Delay_out7"
  Delay8/1 -> hold_fir_tap9/1(data_in), Delay9/1  "xlsub2_Delay_out8"
  Delay10/1 -> hold_fir_tap11/1(data_in), Delay11/1  "xlsub2_Delay_out10"
  Delay12/1 -> hold_fir_tap13/1(data_in), Delay13/1  "xlsub2_Delay_out12"
  en_gen/1(en_out) -> adder_tree/1(en_in), hold_fir_tap15/2(en_in), hold_fir_tap13/2(en_in), hold_fir_tap11/2(en_in), hold_fir_tap9/2(en_in), hold_fir_tap8/2(en_in), hold_fir_tap7/2(en_in), hold_fir_tap5/2(en_in), hold_fir_tap3/2(en_in), hold_fir_tap1/2(en_in)  "en"
  adder_tree/2(dout) -> out/1
  adder_tree/1(en_out) -> en_out/1
  hold_fir_tap15/1(mult_out) -> adder_tree/10(din9)  "hold_fir_tap_out9"
  hold_fir_tap13/1(mult_out) -> adder_tree/9(din8)  "hold_fir_tap_out8"
  hold_fir_tap11/1(mult_out) -> adder_tree/8(din7)  "hold_fir_tap_out7"
  hold_fir_tap9/1(mult_out) -> adder_tree/7(din6)  "hold_fir_tap_out6"
  hold_fir_tap8/1(mult_out) -> adder_tree/6(din5)  "hold_fir_tap_out5"
  hold_fir_tap7/1(mult_out) -> adder_tree/5(din4)  "hold_fir_tap_out4"
  hold_fir_tap5/1(mult_out) -> adder_tree/4(din3)  "hold_fir_tap_out3"
  hold_fir_tap3/1(mult_out) -> adder_tree/3(din2)  "hold_fir_tap_out2"
  hold_fir_tap1/1(mult_out) -> adder_tree/2(din1)  "hold_fir_tap_out1"
  Delay14/1 -> hold_fir_tap15/1(data_in)  "xlsub2_Delay_out14"
  Delay13/1 -> Delay14/1  "xlsub2_Delay_out13"
  Delay11/1 -> Delay12/1  "xlsub2_Delay_out11"
  Delay9/1 -> Delay10/1  "xlsub2_Delay_out9"
  Delay5/1 -> Delay6/1  "xlsub2_Delay_out5"
  Delay3/1 -> Delay4/1  "xlsub2_Delay_out3"
  Delay1/1 -> Delay2/1  "xlsub2_Delay_out1"
  sync/1 -> en_gen/1(sync_in)

== system l8_ver130/s3/cic_pow2_0 ==
  in1 : Inport
  in2 : Inport  Port=2
  in3 : Inport  Port=3
  in4 : Inport  Port=4
  in5 : Inport  Port=5
  in6 : Inport  Port=6
  in7 : Inport  Port=7
  in8 : Inport  Port=8
  in9 : Inport  Port=9
  in10 : Inport  Port=10
  in11 : Inport  Port=11
  in12 : Inport  Port=12
  in13 : Inport  Port=13
  in14 : Inport  Port=14
  in15 : Inport  Port=15
  in16 : Inport  Port=16
  sync_in : Inport  Port=17
  Stage1_dec2 : Subsystem
      settings: AttributesFormatString=|
      | nonrecursive
      | 24_22_in
      | clock(actualy/effective): 1/1
  Stage2_dec2 : Subsystem
      settings: AttributesFormatString=|
      | nonrecursive
      | 28_22_in
      | clock(actualy/effective): 1/1
  Stage3_dec2 : Subsystem
      settings: AttributesFormatString=|
      | nonrecursive
      | 32_22_in
      | clock(actualy/effective): 1/1
  Stage4_dec2 : Subsystem
      settings: AttributesFormatString=|
      | nonrecursive
      | 36_22_in
      | clock(actualy/effective): 1/1
  out1 : Outport
  sync_out : Outport  Port=2
  wires:
  sync_in/1 -> Stage4_dec2/3(sync_in), Stage3_dec2/5(sync_in), Stage2_dec2/9(sync_in), Stage1_dec2/17(sync_in)
  Stage4_dec2/2(sync_out) -> sync_out/1  "stage_sync_out4"
  Stage4_dec2/1(out1) -> out1/1
  Stage3_dec2/2(out2) -> Stage4_dec2/2(in2)  "sig4_2"
  Stage3_dec2/1(out1) -> Stage4_dec2/1(in1)  "sig4_1"
  Stage2_dec2/4(out4) -> Stage3_dec2/4(in4)  "sig3_4"
  Stage2_dec2/3(out3) -> Stage3_dec2/3(in3)  "sig3_3"
  Stage2_dec2/2(out2) -> Stage3_dec2/2(in2)  "sig3_2"
  Stage2_dec2/1(out1) -> Stage3_dec2/1(in1)  "sig3_1"
  Stage1_dec2/8(out8) -> Stage2_dec2/8(in8)  "sig2_8"
  Stage1_dec2/7(out7) -> Stage2_dec2/7(in7)  "sig2_7"
  Stage1_dec2/6(out6) -> Stage2_dec2/6(in6)  "sig2_6"
  Stage1_dec2/5(out5) -> Stage2_dec2/5(in5)  "sig2_5"
  Stage1_dec2/4(out4) -> Stage2_dec2/4(in4)  "sig2_4"
  Stage1_dec2/3(out3) -> Stage2_dec2/3(in3)  "sig2_3"
  Stage1_dec2/2(out2) -> Stage2_dec2/2(in2)  "sig2_2"
  Stage1_dec2/1(out1) -> Stage2_dec2/1(in1)  "sig2_1"
  in16/1 -> Stage1_dec2/16(in16)
  in15/1 -> Stage1_dec2/15(in15)
  in14/1 -> Stage1_dec2/14(in14)
  in13/1 -> Stage1_dec2/13(in13)
  in12/1 -> Stage1_dec2/12(in12)
  in11/1 -> Stage1_dec2/11(in11)
  in10/1 -> Stage1_dec2/10(in10)
  in9/1 -> Stage1_dec2/9(in9)
  in8/1 -> Stage1_dec2/8(in8)
  in7/1 -> Stage1_dec2/7(in7)
  in6/1 -> Stage1_dec2/6(in6)
  in5/1 -> Stage1_dec2/5(in5)
  in4/1 -> Stage1_dec2/4(in4)
  in3/1 -> Stage1_dec2/3(in3)
  in2/1 -> Stage1_dec2/2(in2)
  in1/1 -> Stage1_dec2/1(in1)

== system l8_ver130/s3/cic_pow2_1 ==
  in1 : Inport
  in2 : Inport  Port=2
  in3 : Inport  Port=3
  in4 : Inport  Port=4
  in5 : Inport  Port=5
  in6 : Inport  Port=6
  in7 : Inport  Port=7
  in8 : Inport  Port=8
  in9 : Inport  Port=9
  in10 : Inport  Port=10
  in11 : Inport  Port=11
  in12 : Inport  Port=12
  in13 : Inport  Port=13
  in14 : Inport  Port=14
  in15 : Inport  Port=15
  in16 : Inport  Port=16
  sync_in : Inport  Port=17
  Stage1_dec2 : Subsystem
      settings: AttributesFormatString=|
      | nonrecursive
      | 24_22_in
      | clock(actualy/effective): 1/1
  Stage2_dec2 : Subsystem
      settings: AttributesFormatString=|
      | nonrecursive
      | 28_22_in
      | clock(actualy/effective): 1/1
  Stage3_dec2 : Subsystem
      settings: AttributesFormatString=|
      | nonrecursive
      | 32_22_in
      | clock(actualy/effective): 1/1
  Stage4_dec2 : Subsystem
      settings: AttributesFormatString=|
      | nonrecursive
      | 36_22_in
      | clock(actualy/effective): 1/1
  out1 : Outport
  sync_out : Outport  Port=2
  wires:
  sync_in/1 -> Stage4_dec2/3(sync_in), Stage3_dec2/5(sync_in), Stage2_dec2/9(sync_in), Stage1_dec2/17(sync_in)
  Stage4_dec2/2(sync_out) -> sync_out/1  "stage_sync_out4"
  Stage4_dec2/1(out1) -> out1/1
  Stage3_dec2/2(out2) -> Stage4_dec2/2(in2)  "sig4_2"
  Stage3_dec2/1(out1) -> Stage4_dec2/1(in1)  "sig4_1"
  Stage2_dec2/4(out4) -> Stage3_dec2/4(in4)  "sig3_4"
  Stage2_dec2/3(out3) -> Stage3_dec2/3(in3)  "sig3_3"
  Stage2_dec2/2(out2) -> Stage3_dec2/2(in2)  "sig3_2"
  Stage2_dec2/1(out1) -> Stage3_dec2/1(in1)  "sig3_1"
  Stage1_dec2/8(out8) -> Stage2_dec2/8(in8)  "sig2_8"
  Stage1_dec2/7(out7) -> Stage2_dec2/7(in7)  "sig2_7"
  Stage1_dec2/6(out6) -> Stage2_dec2/6(in6)  "sig2_6"
  Stage1_dec2/5(out5) -> Stage2_dec2/5(in5)  "sig2_5"
  Stage1_dec2/4(out4) -> Stage2_dec2/4(in4)  "sig2_4"
  Stage1_dec2/3(out3) -> Stage2_dec2/3(in3)  "sig2_3"
  Stage1_dec2/2(out2) -> Stage2_dec2/2(in2)  "sig2_2"
  Stage1_dec2/1(out1) -> Stage2_dec2/1(in1)  "sig2_1"
  in16/1 -> Stage1_dec2/16(in16)
  in15/1 -> Stage1_dec2/15(in15)
  in14/1 -> Stage1_dec2/14(in14)
  in13/1 -> Stage1_dec2/13(in13)
  in12/1 -> Stage1_dec2/12(in12)
  in11/1 -> Stage1_dec2/11(in11)
  in10/1 -> Stage1_dec2/10(in10)
  in9/1 -> Stage1_dec2/9(in9)
  in8/1 -> Stage1_dec2/8(in8)
  in7/1 -> Stage1_dec2/7(in7)
  in6/1 -> Stage1_dec2/6(in6)
  in5/1 -> Stage1_dec2/5(in5)
  in4/1 -> Stage1_dec2/4(in4)
  in3/1 -> Stage1_dec2/3(in3)
  in2/1 -> Stage1_dec2/2(in2)
  in1/1 -> Stage1_dec2/1(in1)

== system l8_ver130/s3/cic_pow2_2 ==
  in1 : Inport
  in2 : Inport  Port=2
  in3 : Inport  Port=3
  in4 : Inport  Port=4
  in5 : Inport  Port=5
  in6 : Inport  Port=6
  in7 : Inport  Port=7
  in8 : Inport  Port=8
  in9 : Inport  Port=9
  in10 : Inport  Port=10
  in11 : Inport  Port=11
  in12 : Inport  Port=12
  in13 : Inport  Port=13
  in14 : Inport  Port=14
  in15 : Inport  Port=15
  in16 : Inport  Port=16
  sync_in : Inport  Port=17
  Stage1_dec2 : Subsystem
      settings: AttributesFormatString=|
      | nonrecursive
      | 24_22_in
      | clock(actualy/effective): 1/1
  Stage2_dec2 : Subsystem
      settings: AttributesFormatString=|
      | nonrecursive
      | 28_22_in
      | clock(actualy/effective): 1/1
  Stage3_dec2 : Subsystem
      settings: AttributesFormatString=|
      | nonrecursive
      | 32_22_in
      | clock(actualy/effective): 1/1
  Stage4_dec2 : Subsystem
      settings: AttributesFormatString=|
      | nonrecursive
      | 36_22_in
      | clock(actualy/effective): 1/1
  out1 : Outport
  sync_out : Outport  Port=2
  wires:
  sync_in/1 -> Stage4_dec2/3(sync_in), Stage3_dec2/5(sync_in), Stage2_dec2/9(sync_in), Stage1_dec2/17(sync_in)
  Stage4_dec2/2(sync_out) -> sync_out/1  "stage_sync_out4"
  Stage4_dec2/1(out1) -> out1/1
  Stage3_dec2/2(out2) -> Stage4_dec2/2(in2)  "sig4_2"
  Stage3_dec2/1(out1) -> Stage4_dec2/1(in1)  "sig4_1"
  Stage2_dec2/4(out4) -> Stage3_dec2/4(in4)  "sig3_4"
  Stage2_dec2/3(out3) -> Stage3_dec2/3(in3)  "sig3_3"
  Stage2_dec2/2(out2) -> Stage3_dec2/2(in2)  "sig3_2"
  Stage2_dec2/1(out1) -> Stage3_dec2/1(in1)  "sig3_1"
  Stage1_dec2/8(out8) -> Stage2_dec2/8(in8)  "sig2_8"
  Stage1_dec2/7(out7) -> Stage2_dec2/7(in7)  "sig2_7"
  Stage1_dec2/6(out6) -> Stage2_dec2/6(in6)  "sig2_6"
  Stage1_dec2/5(out5) -> Stage2_dec2/5(in5)  "sig2_5"
  Stage1_dec2/4(out4) -> Stage2_dec2/4(in4)  "sig2_4"
  Stage1_dec2/3(out3) -> Stage2_dec2/3(in3)  "sig2_3"
  Stage1_dec2/2(out2) -> Stage2_dec2/2(in2)  "sig2_2"
  Stage1_dec2/1(out1) -> Stage2_dec2/1(in1)  "sig2_1"
  in16/1 -> Stage1_dec2/16(in16)
  in15/1 -> Stage1_dec2/15(in15)
  in14/1 -> Stage1_dec2/14(in14)
  in13/1 -> Stage1_dec2/13(in13)
  in12/1 -> Stage1_dec2/12(in12)
  in11/1 -> Stage1_dec2/11(in11)
  in10/1 -> Stage1_dec2/10(in10)
  in9/1 -> Stage1_dec2/9(in9)
  in8/1 -> Stage1_dec2/8(in8)
  in7/1 -> Stage1_dec2/7(in7)
  in6/1 -> Stage1_dec2/6(in6)
  in5/1 -> Stage1_dec2/5(in5)
  in4/1 -> Stage1_dec2/4(in4)
  in3/1 -> Stage1_dec2/3(in3)
  in2/1 -> Stage1_dec2/2(in2)
  in1/1 -> Stage1_dec2/1(in1)
